simulink model "HDVSim_BEV_WPT_Truck"  (R2018b)
settings: Solver=ode3  StartTime=0.0  StopTime=inf  FixedStep=sample_time  MaxStep=0.1  RelTol=1e-3  EnableMultiTasking=on
model workspace (MAT data in the model file):
  Anschaffungskosten = [3707764736; 2; 1; 1; 1; 1]
  Bat : struct (value not decoded)
  DrvTrn = 12
  Eigenschaften = [3707764736; 2; 1; 1; 2; 2]
  Electric_Truck = 1
  Elektro_LKW = 1
  Fahrzyklus = 2
  FuelCell : struct (value not decoded)
  Fueltype = 12
  Gewichte = [3707764736; 2; 1; 1; 3; 3]
  Hybrid_LKW = 1
  Hybrid_Truck = 1
  IZ12 = 3600
  Kraftstoff = 12
  SOC_T_EM_completely_available = 0.5
  SOC_addition = 0.4
  SOC_target = 0.85
  Sailing_without_drag_torque = 1
  MCOS : class17 (value not decoded)
  TCO_Anhaenger = 1
  TCO_Trailer = 1
  T_distance_LPS_down = 250
  T_distance_LPS_up = 250
  Temp1 = [1000 1100 1200 1300 1400 1500 1600 1700 1800 1900 2000 2100 ... (41 elements, 1x41)]
  VSim : struct (value not decoded)
  Vehicle = 'BEV_WPT'
  Vorausschauender_Tempomat = 1
  WPT : struct (value not decoded)
  acquisition_costs = [3707764736; 2; 1; 1; 3; 3]
  addition_for_critical_slope = 0.01
  ambient : struct (value not decoded)
  auxiliary_consumers : struct (value not decoded)
  critical_altitude_difference = 50
  cycle : struct (value not decoded)
  dcycle = 2
  distance_1_PPC = 175
  distance_2_PPC = 200
  distance_PPC_altitude_difference = 10000
  driver : struct (value not decoded)
  em : struct (value not decoded)
  em_M_max = 1563
  engine : struct (value not decoded)
  final_drive : struct (value not decoded)
  ifElectric = 1
  ifOptimized = 1
  look_ahead_PPC = 250
  max_distance = 358138
  name = 'Verbrauchssimulation_Elektro_WPT_LKW'
  propertie = [3707764736; 2; 1; 1; 4; 4]
  prospective_cruise_control = 1
  sample_time = 0.1
  tank : struct (value not decoded)
  tires : struct (value not decoded)
  transmission : struct (value not decoded)
  v_PPC_delta = 2.77777777778
  v_max_electrical_drive_only = 13.8888888889
  vehicle : struct (value not decoded)
  vehicle_F_brake_max = 309890.14
  MCOS : class17 (value not decoded)
  x = [1563 13 1 400 10.0500050682 4.34242437682 3 1 0 978 1339 2 ... (15 elements, 1x15)]
values from neighbouring files (symbols this model uses):
  transmission = Transmission_gearing(15.86, 7, 0, 0, final_drive.ratio)    [Parameterizing.m]
symbols that are objects in the model workspace (value not decoded): ambient, auxiliary_consumers, Bat, cycle, driver, em, final_drive, tires, vehicle, WPT
undefined symbols (not defined in the file or in its folder): Ion, Lithium
libraries: simulink x22, powerlib x4, electricdrivelib x1
(part 3 of 3)

== stateflow chart HDVSim_BEV_WPT_Truck/Power electronics and battery/Chart ==
  state Battery
    % Pure battery operation
    during: I_Bat = I_EM_current; I_WPT = 0;
  state LoadingBattery
    % Excess energy to charge the battery
    during: I_Bat = I_EM_current - WPT_I_max;
    I_WPT = WPT_I_max;
  state WPT
    % Pure inductive driving
    during: I_Bat = 0; I_WPT = I_EM_current;
  state Boost
    % Pull power from WPT and battery
    during: I_Bat = WPT_I_max - I_EM_current;
    I_WPT = WPT_I_max;
  (default) -> Battery
  (junction 15) -> Battery
  Battery -> LoadingBattery [order 2] : [WPT_available == 1 && I_EM_current < WPT_I_max]
  Battery -> Boost [order 3] : [WPT_available == 1 && I_EM_current > WPT_I_max]
  Battery -> WPT [order 1] : [WPT_available == 1 && I_EM_current <= WPT_I_max]
  LoadingBattery -> (junction 15) [order 1] : [WPT_available == 0]
  WPT -> (junction 15) [order 1] : [WPT_available == 0]
  Boost -> (junction 15) [order 1] : [WPT_available == 0]
  WPT -> LoadingBattery [order 3] : [WPT_available == 1 && I_EM_current < WPT_I_max && SOC <= SOCTarget]
  LoadingBattery -> WPT [order 3] : [WPT_available == 1 && I_EM_current > WPT_I_max || SOC > SOCTarget]
  Boost -> LoadingBattery [order 2] : [WPT_available == 1 && I_EM_current < WPT_I_max]
  LoadingBattery -> Boost [order 2] : [WPT_available == 1 && I_EM_current > WPT_I_max]
  WPT -> Boost [order 2] : [WPT_available == 1 && I_EM_current > WPT_I_max]
  Boost -> WPT [order 3] : [WPT_available == 1 && I_EM_current <= WPT_I_max]
  data: I_EM_current input; WPT_available input; I_Bat output; I_WPT output; WPT_voltage local =WPT.Voltage; WPT_P_max local =WPT.P_max; WPT_I_max local =WPT.P_max*1000/WPT.Voltage; SOC input; SOCTarget constant double =WPT.SOC_target

== matlab function HDVSim_BEV_WPT_Truck/Transmission/Determine when shifting occurs/Gear change recommendation/recommend_up_calc_bsfc1/MATLAB Function1 ==
  data: n input; g_2 output; M_max input; n_6 input; n_2 input
function g_2 = fcn(n,M_max,n_6,n_2)
%#codegen

g_2 = M_max / (n_6 - n_2) * (n - n_2);

== stateflow chart HDVSim_BEV_WPT_Truck/Active cruise control/Chart1 ==
  state Incline
    entry: Signal_incline = 1;
    exit: Signal_incline = 0;
  state Even
  state Decline
    entry: Signal_decline = 1;
    exit: Signal_decline = 0;
  Even -> Incline [order 2] : [look_ahead_PPC > critical_incline &&...
      | look_ahead_PPC+distance_PPC > critical_incline &&...
      | prospective_cruise_control == 1]
  Incline -> Even : [incline_0 < critical_incline &&...
      | incline_100 < critical_incline &&...
      | incline_200 < critical_incline &&...
      | incline_300 < critical_incline &&...
      | incline_400 < critical_incline &&...
      | incline_500 < critical_incline &&...
      | Distance_covered > look_ahead_PPC+distance_PPC]
  (default) -> Even
  Even -> Decline [order 1] : [(Acceleration_0 +...
      | Acceleration_50 +...
      | Acceleration_100 +...
      | Acceleration_150 +...
      | Acceleration_200)/5 >= -(v_max_cycle/distance_2_PPC*v_PPC_delta) &&...
      | (Acceleration_250 +...
      | Acceleration_300 +...
      | Acceleration_350 +...
      | Acceleration_400 +...
      | Acceleration_450)/5 >= (v_max_cycle/distance_2_PPC*v_PPC_delta) &&...
      | Driver_M > 0 &&...
      | prospective_cruise_control == 1 &&...
      | v_current >= 60/3.6]
  Decline -> Even : [Distance_covered_2 > 450 ||...
      | Driver_B > 0]
  data: critical_incline input; Acceleration_0 input; Acceleration_50 input; Acceleration_100 input; Acceleration_150 input; Acceleration_200 input; Acceleration_250 input; Acceleration_300 input; Acceleration_350 input; Acceleration_400 input; Acceleration_450 input; incline_0 input; incline_100 input; incline_200 input; incline_300 input; incline_400 input; incline_500 input; incline_distance_look_ahead_min input; incline_distance_look_ahead_max input; Driver_M input; Driver_B input; Distance_covered input; Distance_covered_2 input; Signal_incline output double; Signal_decline output double; prospective_cruise_control constant double =preditiveCruiseControl; look_ahead constant double; v_PPC_delta constant double; v_max_cycle constant double; distance constant double; distance_2_PPC constant double; distance_PPC constant double; look_ahead_PPC constant double; v_current input
